simulink model "cmpss_external_mode_am263p"  (R2024b)
settings: Solver=FixedStepAuto  StartTime=0.0  StopTime=5  RelTol=1e-3  EnableMultiTasking=on
libraries: peripheral_library_blocks_am263px x2, simulink x1
settings Model: Creator=a0499511  LastModifiedBy=a1255762
settings OptimizationCC: DefaultParameterBehavior=Inlined  EnhancedBackFolding=on  PassReuseOutputArgsAs=Individual arguments  ZeroExternalMemoryAtStartup=off  ZeroInternalMemoryAtStartup=off  MaxStackSize=64  OptimizeBlockOrder=speed  DifferentSizesBufferReuse=on  OptimizationCustomize=off  EfficientTunableParamExpr=on
settings DebuggingCC: ParameterTunabilityLossMsg=error
settings HardwareCC: ProdBitPerPointer=32  ProdBitPerSizeT=32  ProdBitPerPtrDiffT=32  ProdLargestAtomicInteger=Long  ProdLargestAtomicFloat=Double  ProdWordSize=32  ProdLongLongMode=on  ProdHWDeviceType=ARM Compatible->ARM Cortex
settings SFSimCC: MATLABDynamicMemAlloc=off
settings RTWCC: Description=Embedded Coder  SystemTargetFile=ert.tlc  HardwareBoard=AM263PX LP  TemplateMakefile=ert_default_tmf  Toolchain=TI ARM Clang Compiler AM263Px | gmake
settings CodeAppCC: ForceParamTrailComments=on  IgnoreCustomStorageClasses=off  MaxIdLength=256  OperatorAnnotations=on  SimulinkDataObjDesc=on  SFDataObjDesc=on  InsertBlockDesc=on
settings ERTTargetCC: ExtModeTransport=3  ExtModeStaticAlloc=on  ExtModeMexFile=ext_xcp  ExtModeIntrfLevel=Level2 - Open  ERTCustomFileTemplate=AM263x_codertarget_file_process.tlc
settings ConfigComp: CovBoundaryAbsTol=1.0e-5

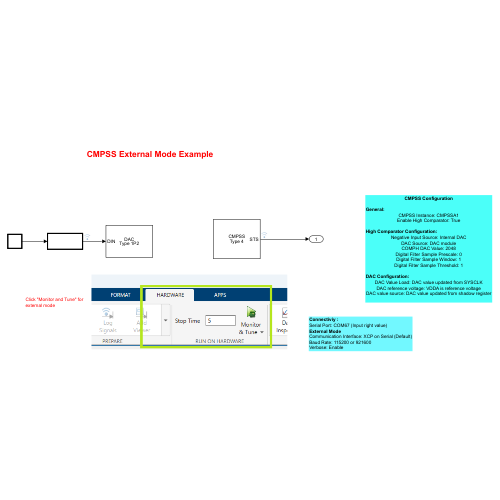
[thumbnail: Simulink's stored preview of the top-level diagram]
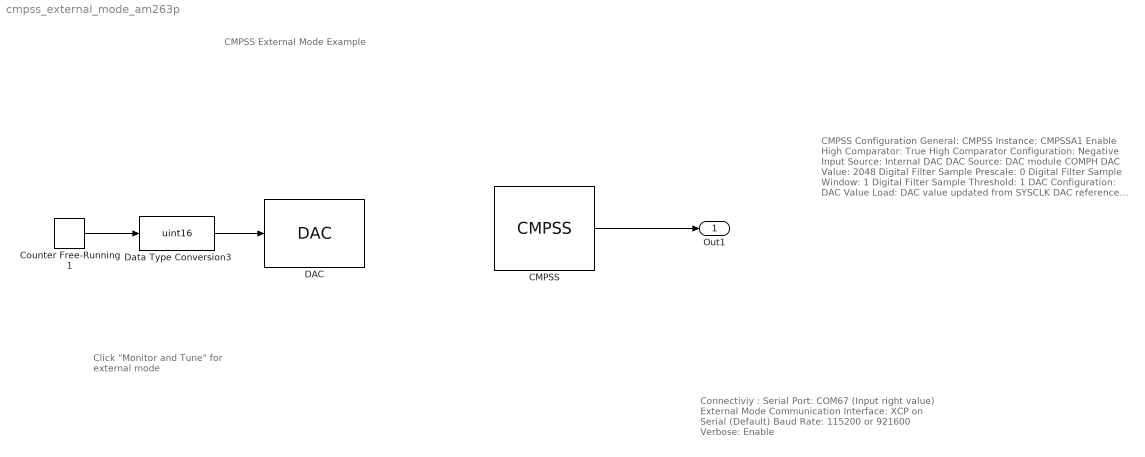
== system cmpss_external_mode_am263p ==
  note: CMPSS External Mode Example
  note: Click "Monitor and Tune" for external mode
  note: Connectiviy :
      | Serial Port: COM67 (Input right value)
      | External Mode
      | Communication Interface: XCP on Serial (Default)
      | Baud Rate: 115200 or 921600
      | Verbose: Enable
  note: CMPSS Configuration
      |
      | General:
      | CMPSS Instance: CMPSSA1
      | Enable High Comparator: True
      |
      | High Comparator Configuration:
      | Negative Input Source: Internal DAC
      | DAC Source: DAC module
      | COMPH DAC Value: 2048
      | Digital Filter Sample Prescale: 0
      | Digital Filter Sample Window: 1
      | Digital Filter Sample Threshold: 1
      |
      | DAC Configuration:
      | DAC Value Load: DAC value updated from SYSCLK
      | DAC reference voltage: VDDA is reference voltage
      | DAC value source: DAC value updated from shadow register
  CMPSS : peripheral_library_blocks_am263px/CMPSS  CMPSS_Instance=CMPSSA1  Enable_Module=on  COMPH_Hysterisis=0 LSB when comparator output is 1  COMPL_Hysterisis=0 LSB when comparator output is 1  Blanking_signal=EPWM1BLANK  Enable_blanking_signal=off  Enable_High_Comparator=on  Enable_Low_Comparator=off  Enable_Latch_Output=off  SampleTime=0.1  Negative_Input_Src=Internal DAC  DAC_Src=DAC module  COMPH_Specify_DAC_params=Dialog  COMPH_DAC1_Initial_Value=0  COMPH_DAC_Value=2048  Invert_COMPH_Output=off  Async_Latch_COMPH=off  CTRIPOUTH=Asynchronous comparator output  CTRIPH=Asynchronous comparator output  Initialize_digital_filter_COMPH=off  Clear_Filter_Latch_COMPH=off  Reset_Latch_EPWMSYNCPER_COMPH=off  Digital_Filter_Sample_Prescale_COMPH=0  Digital_Filter_Sample_Window_COMPH=1  Digital_Filter_Threshold_COMPH=1  Reset_Digital_Filter_Latch_COMPH=off  Max_ramp_generator_ref_value=0  Ramp_gen_decrement_value=0  Ramp_gen_delay_value=0  EPWMSYNCPER=EPWM1SYNCPER  Ramp_gen_reset=Load from shadow register  Positive_Input_Source=External pin INL  COMPL_Specify_DAC_params=Dialog  COMPL_DAC1_Initial_Value=0  COMPL_DAC_Value=0  Output_Inverted_COMPL=off  Asynch_Latch_COMPL=off  Signal_CTRIPOUTL=Asynchronous comparator output  Signal_CTRIPL=Asynchronous comparator output  Initialize_digital_filter_COMPL=off  Clear_Filter_Latch_COMPL=off  Reset_Latch_EPWMSYNCPER_COMPL=off  Digital_Filter_Sample_Prescale_COMPL=0  Digital_Filter_Sample_Window_COMPL=1  Digital_Filter_Threshold_COMPL=1  Reset_Digital_Filter_Latch_COMPL=off  DAC_Value_Load=DAC Value updated from SYSCLK  DAC_Reference_Voltage=VDDA is reference voltage  DAC_Value_Source=DAC value updated from shadow register  Diode_Emulation_Enable=off  Diode_emulation_deactive_source=EPWM0.DEACTIVE  COMPH_DAC_Value2=0  COMPL_DAC_Value2=0
      settings: SourceProductName=Embedded Coder Support Package for AM263PX - Peripheral Blocks
  Counter Free-Running1 : simulink/Sources/Counter Free-Running  NumBits=12  tsamp=-1
  DAC : peripheral_library_blocks_am263px/DAC  Reference_Voltage=VREF reference voltage (External)  Load_Mode=Load on next SYSCLK  EPWMSYNCPER_Signal=EPWM0SYNCPER  Shadow_Value=0  Enable_Output=on  Control_Register=off  Shadow_Register=off  Output_Register=off
      settings: SourceProductName=Embedded Coder Support Package for AM263PX - Peripheral Blocks
  Data Type Conversion3 : DataTypeConversion  OutDataTypeStr=uint16  SaturateOnIntegerOverflow=off  RndMeth=Floor
  Out1 : Outport
  wires:
  Data Type Conversion3/1 -> DAC/1
  Counter Free-Running1/1 -> Data Type Conversion3/1
  CMPSS/1 -> Out1/1
